simulink model "MX_Gateway"  (Simulink 9.0)
settings: Solver=FixedStepDiscrete  StartTime=0.0  StopTime=100  FixedStep=0.01  RelTol=1e-3
values from neighbouring files (symbols this model uses):
  ACTIVE = Bobcat.Parameter; CreateDefine( ACTIVE                         ,1                          ,'Define' ,'defines.h' )    [defines.m]
  ACTIVE_MODE_ONE = Bobcat.Parameter; CreateDefine( ACTIVE_MODE_ONE                ,19                         ,'Define' ,'defines.h' )    [defines.m]
  AUTHENTICATED = Bobcat.Parameter; CreateDefine( AUTHENTICATED                  ,1                          ,'Define' ,'defines.h' )    [defines.m]
  AUX_EMEA_CONTROLS = Bobcat.Parameter; CreateDefine( AUX_EMEA_CONTROLS  ,1   ,'Define' ,'defines.h' )    [enums.m]
  AUX_FLOW_HIGH = Bobcat.Parameter; CreateDefine( AUX_FLOW_HIGH           ,4  ,'Define' ,'defines.h' )    [enums.m]
  AUX_FLOW_INACTIVE = Bobcat.Parameter; CreateDefine( AUX_FLOW_INACTIVE       ,0  ,'Define' ,'defines.h' )    [enums.m]
  AUX_FLOW_LOW = Bobcat.Parameter; CreateDefine( AUX_FLOW_LOW            ,1  ,'Define' ,'defines.h' )    [enums.m]
  AUX_FLOW_MEDIUM = Bobcat.Parameter; CreateDefine( AUX_FLOW_MEDIUM         ,2  ,'Define' ,'defines.h' )    [enums.m]
  AUX_FLOW_STANDARD = Bobcat.Parameter; CreateDefine( AUX_FLOW_STANDARD       ,3  ,'Define' ,'defines.h' )    [enums.m]
  AUX_NON_FUNCTIONAL = Bobcat.Parameter; CreateDefine( AUX_NON_FUNCTIONAL ,255 ,'Define' ,'defines.h' )    [enums.m]
  BASE_ACTIVE = Bobcat.Parameter; CreateDefine( BASE_ACTIVE                    ,253                        ,'Define' ,'defines.h' )    [defines.m]
  BASE_DETENT = Bobcat.Parameter; CreateDefine( BASE_DETENT                    ,1                          ,'Define' ,'defines.h' )    [defines.m]
  BEEP_NONE = Bobcat.Parameter; CreateDefine( BEEP_NONE                      ,255                        ,'Define' ,'defines.h' )    [defines.m]
  CD_AIR_FILTER_DEBOUNCE_NORMAL = Bobcat.Parameter; CreateAliasCD( CD_AIR_FILTER_DEBOUNCE_NORMAL                        ,uint16(500)                ,'ConstantDataPointer' ,'CONSTANT_DATA' ,'AIR_FILTER_DEBOUNCE_NORMAL')    [cdata.m]    (also in hc12_cdata.m; m4_cdata.m)
  CD_AIR_FILTER_DEBOUNCE_NOT_CONNECTED = Bobcat.Parameter; CreateAliasCD( CD_AIR_FILTER_DEBOUNCE_NOT_CONNECTED                 ,uint16(3000)               ,'ConstantDataPointer' ,'CONSTANT_DATA' ,'AIR_FILTER_DEBOUNCE_NOT_CONNECTED')    [cdata.m]    (also in hc12_cdata.m; m4_cdata.m)
  CD_AIR_FILTER_DEBOUNCE_PLUGGED = Bobcat.Parameter; CreateAliasCD( CD_AIR_FILTER_DEBOUNCE_PLUGGED                       ,uint16(3000)               ,'ConstantDataPointer' ,'CONSTANT_DATA' ,'AIR_FILTER_DEBOUNCE_PLUGGED')    [cdata.m]    (also in hc12_cdata.m; m4_cdata.m)
  CD_AIR_FILTER_FUNCTION = Bobcat.Parameter; CreateAliasCD( CD_AIR_FILTER_FUNCTION                               ,uint8(255)                 ,'ConstantDataPointer' ,'CONSTANT_DATA' ,'AIR_FILTER_FUNCTION')    [cdata.m]    (also in hc12_cdata.m; m4_cdata.m)
  CD_AIR_FILTER_PLUGGED_WEIGHT = Bobcat.Parameter; CreateAliasCD( CD_AIR_FILTER_PLUGGED_WEIGHT                         ,uint8(2)                   ,'ConstantDataPointer' ,'CONSTANT_DATA' ,'AIR_FILTER_PLUGGED_WEIGHT')    [cdata.m]    (also in hc12_cdata.m; m4_cdata.m)
  CD_AIR_FILTER_UNPLUGGED_WEIGHT = Bobcat.Parameter; CreateAliasCD( CD_AIR_FILTER_UNPLUGGED_WEIGHT                       ,uint8(1)                   ,'ConstantDataPointer' ,'CONSTANT_DATA' ,'AIR_FILTER_UNPLUGGED_WEIGHT')    [cdata.m]    (also in hc12_cdata.m; m4_cdata.m)
  CD_AUTOIDLE_ARMBAR_DELAY = Bobcat.Parameter; CreateAliasCD( CD_AUTOIDLE_ARMBAR_DELAY                             ,uint8(2)                   ,'ConstantDataPointer' ,'CONSTANT_DATA' ,'AUTOIDLE_ARMBAR_DELAY')    [cdata.m]    (also in hc12_cdata.m; m4_cdata.m)
  CD_AUTOIDLE_FUNCTION = Bobcat.Parameter; CreateAliasCD( CD_AUTOIDLE_FUNCTION                                 ,uint8(255)                 ,'ConstantDataPointer' ,'CONSTANT_DATA' ,'AUTOIDLE_FUNCTION')    [cdata.m]    (also in hc12_cdata.m; m4_cdata.m)
  CD_AUTOIDLE_IDLE_DELAY = Bobcat.Parameter; CreateAliasCD( CD_AUTOIDLE_IDLE_DELAY                               ,uint8(40)                  ,'ConstantDataPointer' ,'CONSTANT_DATA' ,'AUTOIDLE_IDLE_DELAY')    [cdata.m]    (also in hc12_cdata.m; m4_cdata.m)
  CD_AUTOIDLE_LOW_IDLE_START = Bobcat.Parameter; CreateAliasCD( CD_AUTOIDLE_LOW_IDLE_START                           ,uint8(255)                 ,'ConstantDataPointer' ,'CONSTANT_DATA' ,'AUTOIDLE_LOW_IDLE_START')    [cdata.m]    (also in hc12_cdata.m; m4_cdata.m)
  CD_AUTOIDLE_PRESSURE_OFF = Bobcat.Parameter; CreateAliasCD( CD_AUTOIDLE_PRESSURE_OFF                             ,uint16(300)                ,'ConstantDataPointer' ,'CONSTANT_DATA' ,'AUTOIDLE_PRESSURE_OFF')    [cdata.m]    (also in hc12_cdata.m; m4_cdata.m)
  CD_AUTOIDLE_PRESSURE_ON = Bobcat.Parameter; CreateAliasCD( CD_AUTOIDLE_PRESSURE_ON                              ,uint16(270)                ,'ConstantDataPointer' ,'CONSTANT_DATA' ,'AUTOIDLE_PRESSURE_ON')    [cdata.m]    (also in hc12_cdata.m; m4_cdata.m)
  CD_AUTOSHIFT_FUNCTION = Bobcat.Parameter; CreateAliasCD( CD_AUTOSHIFT_FUNCTION                                ,uint8(255)                 ,'ConstantDataPointer' ,'CONSTANT_DATA' ,'AUTOSHIFT_FUNCTION')    [cdata.m]    (also in hc12_cdata.m; m4_cdata.m)
  CD_AUTOSHIFT_PHIGH1 = Bobcat.Parameter; CreateAliasCD( CD_AUTOSHIFT_PHIGH1                                  ,uint16(205)                ,'ConstantDataPointer' ,'CONSTANT_DATA' ,'AUTOSHIFT_PHIGH1')    [cdata.m]    (also in hc12_cdata.m; m4_cdata.m)
  CD_AUTOSHIFT_PHIGH2 = Bobcat.Parameter; CreateAliasCD( CD_AUTOSHIFT_PHIGH2                                  ,uint16(193)                ,'ConstantDataPointer' ,'CONSTANT_DATA' ,'AUTOSHIFT_PHIGH2')    [cdata.m]    (also in hc12_cdata.m; m4_cdata.m)
  CD_AUTOSHIFT_PHIGH_TEMP1 = Bobcat.Parameter; CreateAliasCD( CD_AUTOSHIFT_PHIGH_TEMP1                             ,int16(70)                  ,'ConstantDataPointer' ,'CONSTANT_DATA' ,'AUTOSHIFT_PHIGH_TEMP1')    [cdata.m]    (also in hc12_cdata.m; m4_cdata.m)
  CD_AUTOSHIFT_PHIGH_TEMP2 = Bobcat.Parameter; CreateAliasCD( CD_AUTOSHIFT_PHIGH_TEMP2                             ,int16(160)                 ,'ConstantDataPointer' ,'CONSTANT_DATA' ,'AUTOSHIFT_PHIGH_TEMP2')    [cdata.m]    (also in hc12_cdata.m; m4_cdata.m)
  CD_AUTOSHIFT_PLOW1 = Bobcat.Parameter; CreateAliasCD( CD_AUTOSHIFT_PLOW1                                   ,uint16(130)                ,'ConstantDataPointer' ,'CONSTANT_DATA' ,'AUTOSHIFT_PLOW1')    [cdata.m]    (also in hc12_cdata.m; m4_cdata.m)
  CD_AUTOSHIFT_PLOW2 = Bobcat.Parameter; CreateAliasCD( CD_AUTOSHIFT_PLOW2                                   ,uint16(100)                ,'ConstantDataPointer' ,'CONSTANT_DATA' ,'AUTOSHIFT_PLOW2')    [cdata.m]    (also in hc12_cdata.m; m4_cdata.m)
  CD_AUTOSHIFT_PLOW_TEMP1 = Bobcat.Parameter; CreateAliasCD( CD_AUTOSHIFT_PLOW_TEMP1                              ,int16(20)                  ,'ConstantDataPointer' ,'CONSTANT_DATA' ,'AUTOSHIFT_PLOW_TEMP1')    [cdata.m]    (also in hc12_cdata.m; m4_cdata.m)
  CD_AUTOSHIFT_PLOW_TEMP2 = Bobcat.Parameter; CreateAliasCD( CD_AUTOSHIFT_PLOW_TEMP2                              ,int16(80)                  ,'ConstantDataPointer' ,'CONSTANT_DATA' ,'AUTOSHIFT_PLOW_TEMP2')    [cdata.m]    (also in hc12_cdata.m; m4_cdata.m)
  CD_AUTOSHIFT_TRAVEL_DELAY = Bobcat.Parameter; CreateAliasCD( CD_AUTOSHIFT_TRAVEL_DELAY                            ,uint8(32)                  ,'ConstantDataPointer' ,'CONSTANT_DATA' ,'AUTOSHIFT_TRAVEL_DELAY')    [cdata.m]    (also in hc12_cdata.m; m4_cdata.m)
  CD_AUX2_CRACKPOINT_CURRENT = Bobcat.Parameter; CreateAliasCD( CD_AUX2_CRACKPOINT_CURRENT                           ,fi(0,0,16,12,'hex','0c28') ,'ConstantDataPointer' ,'CONSTANT_DATA' ,'AUX2_CRACKPOINT_CURRENT')    [cdata.m]    (also in hc12_cdata.m; m4_cdata.m)
  CD_AUX2_FLOW_LOW_FUNCTION = Bobcat.Parameter; CreateAliasCD( CD_AUX2_FLOW_LOW_FUNCTION                            ,uint8(255)                 ,'ConstantDataPointer' ,'CONSTANT_DATA' ,'AUX2_FLOW_LOW_FUNCTION')    [cdata.m]    (also in hc12_cdata.m; m4_cdata.m)
  CD_AUX2_FLOW_MED_FUNCTION = Bobcat.Parameter; CreateAliasCD( CD_AUX2_FLOW_MED_FUNCTION                            ,uint8(255)                 ,'ConstantDataPointer' ,'CONSTANT_DATA' ,'AUX2_FLOW_MED_FUNCTION')    [cdata.m]    (also in hc12_cdata.m; m4_cdata.m)
  CD_AUX2_HI_FLOW_PERCENT = Bobcat.Parameter; CreateAliasCD( CD_AUX2_HI_FLOW_PERCENT                              ,fi(0,0,16,16,'hex','ffff') ,'ConstantDataPointer' ,'CONSTANT_DATA' ,'AUX2_HI_FLOW_PERCENT')    [cdata.m]    (also in hc12_cdata.m; m4_cdata.m)
  CD_AUX2_LOW_FLOW_PERCENT = Bobcat.Parameter; CreateAliasCD( CD_AUX2_LOW_FLOW_PERCENT                             ,fi(0,0,16,16,'hex','7333') ,'ConstantDataPointer' ,'CONSTANT_DATA' ,'AUX2_LOW_FLOW_PERCENT')    [cdata.m]    (also in hc12_cdata.m; m4_cdata.m)
  CD_AUX2_MAX_FLOW_CURRENT = Bobcat.Parameter; CreateAliasCD( CD_AUX2_MAX_FLOW_CURRENT                             ,fi(0,0,16,12,'hex','14cc') ,'ConstantDataPointer' ,'CONSTANT_DATA' ,'AUX2_MAX_FLOW_CURRENT')    [cdata.m]    (also in hc12_cdata.m; m4_cdata.m)
  CD_AUX2_MED_FLOW_PERCENT = Bobcat.Parameter; CreateAliasCD( CD_AUX2_MED_FLOW_PERCENT                             ,fi(0,0,16,16,'hex','9999') ,'ConstantDataPointer' ,'CONSTANT_DATA' ,'AUX2_MED_FLOW_PERCENT')    [cdata.m]    (also in hc12_cdata.m; m4_cdata.m)
  CD_AUX_CRACKPOINT_CURRENT = Bobcat.Parameter; CreateAliasCD( CD_AUX_CRACKPOINT_CURRENT                            ,fi(0,0,16,12,'hex','0000') ,'ConstantDataPointer' ,'CONSTANT_DATA' ,'AUX_CRACKPOINT_CURRENT')    [cdata.m]    (also in hc12_cdata.m; m4_cdata.m)
  CD_AUX_DETENT_FLASH_TIME = Bobcat.Parameter; CreateAliasCD( CD_AUX_DETENT_FLASH_TIME                             ,uint16(72)                 ,'ConstantDataPointer' ,'CONSTANT_DATA' ,'AUX_DETENT_FLASH_TIME')    [cdata.m]    (also in hc12_cdata.m; m4_cdata.m)
  CD_AUX_DETENT_HOLD_TIME = Bobcat.Parameter; CreateAliasCD( CD_AUX_DETENT_HOLD_TIME                              ,uint16(100)                ,'ConstantDataPointer' ,'CONSTANT_DATA' ,'AUX_DETENT_HOLD_TIME')    [cdata.m]    (also in hc12_cdata.m; m4_cdata.m)
  CD_AUX_FULL_ON_AUX1 = Bobcat.Parameter; CreateAliasCD( CD_AUX_FULL_ON_AUX1                                  ,fi(0,0,16,12,'hex','10cd') ,'ConstantDataPointer' ,'CONSTANT_DATA' ,'AUX_FULL_ON_AUX1')    [cdata.m]    (also in hc12_cdata.m; m4_cdata.m)
  CD_AUX_FULL_ON_AUX2 = Bobcat.Parameter; CreateAliasCD( CD_AUX_FULL_ON_AUX2                                  ,fi(0,0,16,12,'hex','139d') ,'ConstantDataPointer' ,'CONSTANT_DATA' ,'AUX_FULL_ON_AUX2')    [cdata.m]    (also in hc12_cdata.m; m4_cdata.m)
  CD_AUX_FULL_ON_AUX3 = Bobcat.Parameter; CreateAliasCD( CD_AUX_FULL_ON_AUX3                                  ,fi(0,0,16,12,'hex','17d7') ,'ConstantDataPointer' ,'CONSTANT_DATA' ,'AUX_FULL_ON_AUX3')    [cdata.m]    (also in hc12_cdata.m; m4_cdata.m)
  CD_AUX_FUNCTION = Bobcat.Parameter; CreateAliasCD( CD_AUX_FUNCTION                                      ,uint8(0)                   ,'ConstantDataPointer' ,'CONSTANT_DATA' ,'AUX_FUNCTION')    [cdata.m]    (also in hc12_cdata.m; m4_cdata.m)
  CD_AUX_RATE_LIMIT_CRACKPOINT = Bobcat.Parameter; CreateAliasCD( CD_AUX_RATE_LIMIT_CRACKPOINT                         ,fi(0,0,16,12,'hex','0800') ,'ConstantDataPointer' ,'CONSTANT_DATA' ,'AUX_RATE_LIMIT_CRACKPOINT')    [cdata.m]    (also in hc12_cdata.m; m4_cdata.m)
  CD_AUX_RATE_LIMIT_CRACKPOINT_RATE = Bobcat.Parameter; CreateAliasCD( CD_AUX_RATE_LIMIT_CRACKPOINT_RATE                    ,fi(0,1,32,12,'int',102400) ,'ConstantDataPointer' ,'CONSTANT_DATA' ,'AUX_RATE_LIMIT_CRACKPOINT_RATE')    [cdata.m]    (also in hc12_cdata.m; m4_cdata.m)
  CD_AUX_RATE_LIMIT_FUNCTION = Bobcat.Parameter; CreateAliasCD( CD_AUX_RATE_LIMIT_FUNCTION                           ,uint8(255)                 ,'ConstantDataPointer' ,'CONSTANT_DATA' ,'AUX_RATE_LIMIT_FUNCTION')    [cdata.m]    (also in hc12_cdata.m; m4_cdata.m)
  CD_AUX_RATE_LIMIT_MAX_ENG_SPD = Bobcat.Parameter; CreateAliasCD( CD_AUX_RATE_LIMIT_MAX_ENG_SPD                        ,uint16(1600)               ,'ConstantDataPointer' ,'CONSTANT_DATA' ,'AUX_RATE_LIMIT_MAX_ENG_SPD')    [cdata.m]    (also in hc12_cdata.m; m4_cdata.m)
  CD_AUX_RATE_LIMIT_MIN_ENG_SPD = Bobcat.Parameter; CreateAliasCD( CD_AUX_RATE_LIMIT_MIN_ENG_SPD                        ,uint16(100)                ,'ConstantDataPointer' ,'CONSTANT_DATA' ,'AUX_RATE_LIMIT_MIN_ENG_SPD')    [cdata.m]    (also in hc12_cdata.m; m4_cdata.m)
  CD_AUX_RATE_LIMIT_NORMAL_RATE = Bobcat.Parameter; CreateAliasCD( CD_AUX_RATE_LIMIT_NORMAL_RATE                        ,fi(0,1,32,12,'int',2048)   ,'ConstantDataPointer' ,'CONSTANT_DATA' ,'AUX_RATE_LIMIT_NORMAL_RATE')    [cdata.m]    (also in hc12_cdata.m; m4_cdata.m)
  CD_AUXPOT_CAL_DEADBAND_HIGH = Bobcat.Parameter; CreateAliasCD( CD_AUXPOT_CAL_DEADBAND_HIGH                          ,uint16(574)                ,'ConstantDataPointer' ,'CONSTANT_DATA' ,'AUXPOT_CAL_DEADBAND_HIGH')    [cdata.m]    (also in hc12_cdata.m; m4_cdata.m)
  CD_AUXPOT_CAL_DEADBAND_LOW = Bobcat.Parameter; CreateAliasCD( CD_AUXPOT_CAL_DEADBAND_LOW                           ,uint16(424)                ,'ConstantDataPointer' ,'CONSTANT_DATA' ,'AUXPOT_CAL_DEADBAND_LOW')    [cdata.m]    (also in hc12_cdata.m; m4_cdata.m)
  CD_AUXPOT_CENTER_RATIO = Bobcat.Parameter; CreateAliasCD( CD_AUXPOT_CENTER_RATIO                               ,uint16(500)                ,'ConstantDataPointer' ,'CONSTANT_DATA' ,'AUXPOT_CENTER_RATIO')    [cdata.m]    (also in hc12_cdata.m; m4_cdata.m)
  CD_AUXPOT_DEADBAND = Bobcat.Parameter; CreateAliasCD( CD_AUXPOT_DEADBAND                                   ,uint8(38)                  ,'ConstantDataPointer' ,'CONSTANT_DATA' ,'AUXPOT_DEADBAND')    [cdata.m]    (also in hc12_cdata.m; m4_cdata.m)
  CD_AUXPOT_FUNCTION = Bobcat.Parameter; CreateAliasCD( CD_AUXPOT_FUNCTION                                   ,uint8(0)                   ,'ConstantDataPointer' ,'CONSTANT_DATA' ,'AUXPOT_FUNCTION')    [cdata.m]    (also in hc12_cdata.m; m4_cdata.m)
  CD_AUXPOT_RATIO_GAIN = Bobcat.Parameter; CreateAliasCD( CD_AUXPOT_RATIO_GAIN                                 ,uint16(1000)               ,'ConstantDataPointer' ,'CONSTANT_DATA' ,'AUXPOT_RATIO_GAIN')    [cdata.m]    (also in hc12_cdata.m; m4_cdata.m)
  CD_CAN_THROTTLE_FUNCTION = Bobcat.Parameter; CreateAliasCD( CD_CAN_THROTTLE_FUNCTION                             ,uint8(255)                 ,'ConstantDataPointer' ,'CONSTANT_DATA' ,'CAN_THROTTLE_FUNCTION')    [cdata.m]    (also in hc12_cdata.m; m4_cdata.m)
  CD_CAN_THROTTLE_REVERSE = Bobcat.Parameter; CreateAliasCD( CD_CAN_THROTTLE_REVERSE                              ,uint8(255)                 ,'ConstantDataPointer' ,'CONSTANT_DATA' ,'CAN_THROTTLE_REVERSE')    [cdata.m]    (also in hc12_cdata.m; m4_cdata.m)
  CD_CONSOLE_DEBOUNCE_ABSENT = Bobcat.Parameter; CreateAliasCD( CD_CONSOLE_DEBOUNCE_ABSENT                           ,uint16(12)                 ,'ConstantDataPointer' ,'CONSTANT_DATA' ,'CONSOLE_DEBOUNCE_ABSENT')    [cdata.m]    (also in hc12_cdata.m; m4_cdata.m)
  CD_CONSOLE_DEBOUNCE_OORH = Bobcat.Parameter; CreateAliasCD( CD_CONSOLE_DEBOUNCE_OORH                             ,uint16(12)                 ,'ConstantDataPointer' ,'CONSTANT_DATA' ,'CONSOLE_DEBOUNCE_OORH')    [cdata.m]    (also in hc12_cdata.m; m4_cdata.m)
  CD_CONSOLE_DEBOUNCE_OORL = Bobcat.Parameter; CreateAliasCD( CD_CONSOLE_DEBOUNCE_OORL                             ,uint16(12)                 ,'ConstantDataPointer' ,'CONSTANT_DATA' ,'CONSOLE_DEBOUNCE_OORL')    [cdata.m]    (also in hc12_cdata.m; m4_cdata.m)
  CD_CONSOLE_DEBOUNCE_PRESENT = Bobcat.Parameter; CreateAliasCD( CD_CONSOLE_DEBOUNCE_PRESENT                          ,uint16(12)                 ,'ConstantDataPointer' ,'CONSTANT_DATA' ,'CONSOLE_DEBOUNCE_PRESENT')    [cdata.m]    (also in hc12_cdata.m; m4_cdata.m)
  CD_CONSOLE_FUNCTION = Bobcat.Parameter; CreateAliasCD( CD_CONSOLE_FUNCTION                                  ,uint8(0)                   ,'ConstantDataPointer' ,'CONSTANT_DATA' ,'CONSOLE_FUNCTION')    [cdata.m]    (also in hc12_cdata.m; m4_cdata.m)
  CD_CONSOLE_OORH = Bobcat.Parameter; CreateAliasCD( CD_CONSOLE_OORH                                      ,uint16(740)                ,'ConstantDataPointer' ,'CONSTANT_DATA' ,'CONSOLE_OORH')    [cdata.m]    (also in hc12_cdata.m; m4_cdata.m)
  CD_CONSOLE_OORL = Bobcat.Parameter; CreateAliasCD( CD_CONSOLE_OORL                                      ,uint16(10)                 ,'ConstantDataPointer' ,'CONSTANT_DATA' ,'CONSOLE_OORL')    [cdata.m]    (also in hc12_cdata.m; m4_cdata.m)
  CD_CONSOLE_PRESENT_HIGH = Bobcat.Parameter; CreateAliasCD( CD_CONSOLE_PRESENT_HIGH                              ,uint16(515)                ,'ConstantDataPointer' ,'CONSTANT_DATA' ,'CONSOLE_PRESENT_HIGH')    [cdata.m]    (also in hc12_cdata.m; m4_cdata.m)
  CD_CONSOLE_PRESENT_LOW = Bobcat.Parameter; CreateAliasCD( CD_CONSOLE_PRESENT_LOW                               ,uint16(375)                ,'ConstantDataPointer' ,'CONSTANT_DATA' ,'CONSOLE_PRESENT_LOW')    [cdata.m]    (also in hc12_cdata.m; m4_cdata.m)
  CD_DELUXE_GEN5_5_FUNCTION = Bobcat.Parameter; CreateAliasCD( CD_DELUXE_GEN5_5_FUNCTION                            ,uint8(255)                 ,'ConstantDataPointer' ,'CONSTANT_DATA' ,'DELUXE_GEN5_5_FUNCTION')    [cdata.m]    (also in hc12_cdata.m; m4_cdata.m)
  CD_DELUXE_GEN5_FUNCTION = Bobcat.Parameter; CreateAliasCD( CD_DELUXE_GEN5_FUNCTION                              ,uint8(255)                 ,'ConstantDataPointer' ,'CONSTANT_DATA' ,'DELUXE_GEN5_FUNCTION')    [cdata.m]    (also in hc12_cdata.m; m4_cdata.m)
  CD_DERATE_ENGINE_SPEED_DEBOUNCE_HIGH = Bobcat.Parameter; CreateAliasCD( CD_DERATE_ENGINE_SPEED_DEBOUNCE_HIGH                 ,uint16(200)                ,'ConstantDataPointer' ,'CONSTANT_DATA' ,'DERATE_ENGINE_SPEED_DEBOUNCE_HIGH')    [cdata.m]    (also in hc12_cdata.m; m4_cdata.m)
  CD_DERATE_ENGINE_SPEED_DEBOUNCE_SHUTDOWN = Bobcat.Parameter; CreateAliasCD( CD_DERATE_ENGINE_SPEED_DEBOUNCE_SHUTDOWN             ,uint16(1000)               ,'ConstantDataPointer' ,'CONSTANT_DATA' ,'DERATE_ENGINE_SPEED_DEBOUNCE_SHUTDOWN')    [cdata.m]    (also in hc12_cdata.m; m4_cdata.m)
  CD_DERATE_ENGINE_SPEED_HIGH_OFFSET = Bobcat.Parameter; CreateAliasCD( CD_DERATE_ENGINE_SPEED_HIGH_OFFSET                   ,uint16(200)                ,'ConstantDataPointer' ,'CONSTANT_DATA' ,'DERATE_ENGINE_SPEED_HIGH_OFFSET')    [cdata.m]    (also in hc12_cdata.m; m4_cdata.m)
  CD_DERATE_METHOD = Bobcat.Parameter; CreateAliasCD( CD_DERATE_METHOD                                     ,uint8(0)                   ,'ConstantDataPointer' ,'CONSTANT_DATA' ,'DERATE_METHOD')    [cdata.m]    (also in hc12_cdata.m; m4_cdata.m)
  CD_DUMP_VALVE_FUNCTION = Bobcat.Parameter; CreateAliasCD( CD_DUMP_VALVE_FUNCTION                               ,uint8(255)                 ,'ConstantDataPointer' ,'CONSTANT_DATA' ,'DUMP_VALVE_FUNCTION')    [cdata.m]    (also in hc12_cdata.m; m4_cdata.m)
  CD_DUMP_VALVE_ON_CURRENT = Bobcat.Parameter; CreateAliasCD( CD_DUMP_VALVE_ON_CURRENT                             ,fi(0,0,16,12,'hex','ffff') ,'ConstantDataPointer' ,'CONSTANT_DATA' ,'DUMP_VALVE_ON_CURRENT')    [cdata.m]    (also in hc12_cdata.m; m4_cdata.m)
  CD_ECO_MODE_THROTTLE_REDUCTION = Bobcat.Parameter; CreateAliasCD( CD_ECO_MODE_THROTTLE_REDUCTION                       ,uint16(850)                ,'ConstantDataPointer' ,'CONSTANT_DATA' ,'ECO_MODE_THROTTLE_REDUCTION')    [cdata.m]    (also in hc12_cdata.m; m4_cdata.m)
  CD_ECT_FILTER_GAIN = Bobcat.Parameter; CreateAliasCD( CD_ECT_FILTER_GAIN                                   ,uint8(6)                   ,'ConstantDataPointer' ,'CONSTANT_DATA' ,'ECT_FILTER_GAIN')    [cdata.m]    (also in hc12_cdata.m; m4_cdata.m)
  CD_ECU_FUNCTION = Bobcat.Parameter; CreateAliasCD( CD_ECU_FUNCTION                                      ,uint8(255)                 ,'ConstantDataPointer' ,'CONSTANT_DATA' ,'ECU_FUNCTION')    [cdata.m]    (also in hc12_cdata.m; m4_cdata.m)
  CD_ECU_HOLD_DEBOUNCE_ERROR_ON = Bobcat.Parameter; CreateAliasCD( CD_ECU_HOLD_DEBOUNCE_ERROR_ON                        ,uint16(20)                 ,'ConstantDataPointer' ,'CONSTANT_DATA' ,'ECU_HOLD_DEBOUNCE_ERROR_ON')    [cdata.m]    (also in hc12_cdata.m; m4_cdata.m)
  CD_EMEA_CONTROLS_HOLD_TIME = Bobcat.Parameter; CreateAliasCD( CD_EMEA_CONTROLS_HOLD_TIME                           ,uint16(30)                 ,'ConstantDataPointer' ,'CONSTANT_DATA' ,'EMEA_CONTROLS_HOLD_TIME')    [cdata.m]    (also in hc12_cdata.m; m4_cdata.m)
  CD_EMEA_CONTROLS_OPTION_A = Bobcat.Parameter; CreateAliasCD( CD_EMEA_CONTROLS_OPTION_A                            ,uint8(255)                 ,'ConstantDataPointer' ,'CONSTANT_DATA' ,'EMEA_CONTROLS_OPTION_A')    [cdata.m]    (also in hc12_cdata.m; m4_cdata.m)
  CD_EMEA_CONTROLS_OPTION_C = Bobcat.Parameter; CreateAliasCD( CD_EMEA_CONTROLS_OPTION_C                            ,uint8(255)                 ,'ConstantDataPointer' ,'CONSTANT_DATA' ,'EMEA_CONTROLS_OPTION_C')    [cdata.m]    (also in hc12_cdata.m; m4_cdata.m)
  CD_EMEA_CONTROLS_REVERSE = Bobcat.Parameter; CreateAliasCD( CD_EMEA_CONTROLS_REVERSE                             ,uint8(255)                 ,'ConstantDataPointer' ,'CONSTANT_DATA' ,'EMEA_CONTROLS_REVERSE')    [cdata.m]    (also in hc12_cdata.m; m4_cdata.m)
  CD_EMEA_ELECTRIC_VERSION = Bobcat.Parameter; CreateAliasCD( CD_EMEA_ELECTRIC_VERSION                             ,uint8(0)                   ,'ConstantDataPointer' ,'CONSTANT_DATA' ,'EMEA_ELECTRIC_VERSION')    [cdata.m]    (also in hc12_cdata.m; m4_cdata.m)
  CD_EMEA_POT_CAL_DEADBAND_HIGH = Bobcat.Parameter; CreateAliasCD( CD_EMEA_POT_CAL_DEADBAND_HIGH                        ,uint16(574)                ,'ConstantDataPointer' ,'CONSTANT_DATA' ,'EMEA_POT_CAL_DEADBAND_HIGH')    [cdata.m]    (also in hc12_cdata.m; m4_cdata.m)
  CD_EMEA_POT_CAL_DEADBAND_LOW = Bobcat.Parameter; CreateAliasCD( CD_EMEA_POT_CAL_DEADBAND_LOW                         ,uint16(424)                ,'ConstantDataPointer' ,'CONSTANT_DATA' ,'EMEA_POT_CAL_DEADBAND_LOW')    [cdata.m]    (also in hc12_cdata.m; m4_cdata.m)
  CD_ENGINE_RPM_FACTOR = Bobcat.Parameter; CreateAliasCD( CD_ENGINE_RPM_FACTOR                                 ,uint8(35)                  ,'ConstantDataPointer' ,'CONSTANT_DATA' ,'ENGINE_RPM_FACTOR')    [cdata.m]    (also in hc12_cdata.m; m4_cdata.m)
  CD_ENGINE_SPEED_FILTER_FAST_VALUE = Bobcat.Parameter; CreateAliasCD( CD_ENGINE_SPEED_FILTER_FAST_VALUE                    ,uint8(1)                   ,'ConstantDataPointer' ,'CONSTANT_DATA' ,'ENGINE_SPEED_FILTER_FAST_VALUE')    [cdata.m]    (also in hc12_cdata.m; m4_cdata.m)
  CD_ENGINE_SPEED_FILTER_SLOW_VALUE = Bobcat.Parameter; CreateAliasCD( CD_ENGINE_SPEED_FILTER_SLOW_VALUE                    ,uint8(4)                   ,'ConstantDataPointer' ,'CONSTANT_DATA' ,'ENGINE_SPEED_FILTER_SLOW_VALUE')    [cdata.m]    (also in hc12_cdata.m; m4_cdata.m)
  CD_ENGINE_SPEED_RUNNING = Bobcat.Parameter; CreateAliasCD( CD_ENGINE_SPEED_RUNNING                              ,uint16(800)                ,'ConstantDataPointer' ,'CONSTANT_DATA' ,'ENGINE_SPEED_RUNNING')    [cdata.m]    (also in hc12_cdata.m; m4_cdata.m)
  CD_ENGINE_START_DELAY_DEBOUNCE_RPM_NO_SIGNAL = Bobcat.Parameter; CreateAliasCD( CD_ENGINE_START_DELAY_DEBOUNCE_RPM_NO_SIGNAL         ,uint16(0)                  ,'ConstantDataPointer' ,'CONSTANT_DATA' ,'ENGINE_START_DELAY_DEBOUNCE_RPM_NO_SIGNAL')    [cdata.m]    (also in hc12_cdata.m; m4_cdata.m)
  CD_ENGINE_START_DELAY_OFF_TIME = Bobcat.Parameter; CreateAliasCD( CD_ENGINE_START_DELAY_OFF_TIME                       ,uint16(0)                  ,'ConstantDataPointer' ,'CONSTANT_DATA' ,'ENGINE_START_DELAY_OFF_TIME')    [cdata.m]    (also in hc12_cdata.m; m4_cdata.m)
  CD_ENGINE_START_DELAY_RPM_NO_SIGNAL = Bobcat.Parameter; CreateAliasCD( CD_ENGINE_START_DELAY_RPM_NO_SIGNAL                  ,uint16(0)                  ,'ConstantDataPointer' ,'CONSTANT_DATA' ,'ENGINE_START_DELAY_RPM_NO_SIGNAL')    [cdata.m]    (also in hc12_cdata.m; m4_cdata.m)
  CD_EOP_DEBOUNCE_EXTREMELY_LOW = Bobcat.Parameter; CreateAliasCD( CD_EOP_DEBOUNCE_EXTREMELY_LOW                        ,uint16(50)                 ,'ConstantDataPointer' ,'CONSTANT_DATA' ,'EOP_DEBOUNCE_EXTREMELY_LOW')    [cdata.m]    (also in hc12_cdata.m; m4_cdata.m)
  CD_EOP_DEBOUNCE_NORMAL = Bobcat.Parameter; CreateAliasCD( CD_EOP_DEBOUNCE_NORMAL                               ,uint16(50)                 ,'ConstantDataPointer' ,'CONSTANT_DATA' ,'EOP_DEBOUNCE_NORMAL')    [cdata.m]    (also in hc12_cdata.m; m4_cdata.m)
  CD_EOP_DEBOUNCE_SHUTDOWN = Bobcat.Parameter; CreateAliasCD( CD_EOP_DEBOUNCE_SHUTDOWN                             ,uint16(100)                ,'ConstantDataPointer' ,'CONSTANT_DATA' ,'EOP_DEBOUNCE_SHUTDOWN')    [cdata.m]    (also in hc12_cdata.m; m4_cdata.m)
  CD_EOP_FILTER_VALUE = Bobcat.Parameter; CreateAliasCD( CD_EOP_FILTER_VALUE                                  ,uint8(3)                   ,'ConstantDataPointer' ,'CONSTANT_DATA' ,'EOP_FILTER_VALUE')    [cdata.m]    (also in hc12_cdata.m; m4_cdata.m)
  CD_EOP_FUNCTION = Bobcat.Parameter; CreateAliasCD( CD_EOP_FUNCTION                                      ,uint8(1)                   ,'ConstantDataPointer' ,'CONSTANT_DATA' ,'EOP_FUNCTION')    [cdata.m]    (also in hc12_cdata.m; m4_cdata.m)
  CD_EOP_SWITCH_GAUGE_VALUE = Bobcat.Parameter; CreateAliasCD( CD_EOP_SWITCH_GAUGE_VALUE                            ,uint16(125)                ,'ConstantDataPointer' ,'CONSTANT_DATA' ,'EOP_SWITCH_GAUGE_VALUE')    [cdata.m]    (also in hc12_cdata.m; m4_cdata.m)
  CD_EOP_SWITCH_LOW = Bobcat.Parameter; CreateAliasCD( CD_EOP_SWITCH_LOW                                    ,uint16(50)                 ,'ConstantDataPointer' ,'CONSTANT_DATA' ,'EOP_SWITCH_LOW')    [cdata.m]    (also in hc12_cdata.m; m4_cdata.m)
  CD_EOP_SWITCH_PRESSURE_VALUE = Bobcat.Parameter; CreateAliasCD( CD_EOP_SWITCH_PRESSURE_VALUE                         ,uint16(40)                 ,'ConstantDataPointer' ,'CONSTANT_DATA' ,'EOP_SWITCH_PRESSURE_VALUE')    [cdata.m]    (also in hc12_cdata.m; m4_cdata.m)
  CD_EOT_DEBOUNCE_EXTREMELY_LOW = Bobcat.Parameter; CreateAliasCD( CD_EOT_DEBOUNCE_EXTREMELY_LOW                        ,uint16(2)                  ,'ConstantDataPointer' ,'CONSTANT_DATA' ,'EOT_DEBOUNCE_EXTREMELY_LOW')    [cdata.m]    (also in hc12_cdata.m; m4_cdata.m)
  CD_EOT_DEBOUNCE_LOW = Bobcat.Parameter; CreateAliasCD( CD_EOT_DEBOUNCE_LOW                                  ,uint16(2)                  ,'ConstantDataPointer' ,'CONSTANT_DATA' ,'EOT_DEBOUNCE_LOW')    [cdata.m]    (also in hc12_cdata.m; m4_cdata.m)
  CD_EOT_DEBOUNCE_NORMAL = Bobcat.Parameter; CreateAliasCD( CD_EOT_DEBOUNCE_NORMAL                               ,uint16(2)                  ,'ConstantDataPointer' ,'CONSTANT_DATA' ,'EOT_DEBOUNCE_NORMAL')    [cdata.m]    (also in hc12_cdata.m; m4_cdata.m)
  CD_EOT_EXTREMELY_LOW = Bobcat.Parameter; CreateAliasCD( CD_EOT_EXTREMELY_LOW                                 ,uint16(81)                 ,'ConstantDataPointer' ,'CONSTANT_DATA' ,'EOT_EXTREMELY_LOW')    [cdata.m]    (also in hc12_cdata.m; m4_cdata.m)
  CD_EOT_FUNCTION = Bobcat.Parameter; CreateAliasCD( CD_EOT_FUNCTION                                      ,uint8(255)                 ,'ConstantDataPointer' ,'CONSTANT_DATA' ,'EOT_FUNCTION')    [cdata.m]    (also in hc12_cdata.m; m4_cdata.m)
  CD_EOT_LOW = Bobcat.Parameter; CreateAliasCD( CD_EOT_LOW                                           ,uint16(90)                 ,'ConstantDataPointer' ,'CONSTANT_DATA' ,'EOT_LOW')    [cdata.m]    (also in hc12_cdata.m; m4_cdata.m)
  CD_EOT_TEMPERATURE_HYSTERESIS = Bobcat.Parameter; CreateAliasCD( CD_EOT_TEMPERATURE_HYSTERESIS                        ,uint16(2)                  ,'ConstantDataPointer' ,'CONSTANT_DATA' ,'EOT_TEMPERATURE_HYSTERESIS')    [cdata.m]    (also in hc12_cdata.m; m4_cdata.m)
  CD_FAST_TRAVEL_FUNCTION = Bobcat.Parameter; CreateAliasCD( CD_FAST_TRAVEL_FUNCTION                              ,uint8(255)                 ,'ConstantDataPointer' ,'CONSTANT_DATA' ,'FAST_TRAVEL_FUNCTION')    [cdata.m]    (also in hc12_cdata.m; m4_cdata.m)
  CD_FAST_TRAVEL_THROTTLE_DELAY = Bobcat.Parameter; CreateAliasCD( CD_FAST_TRAVEL_THROTTLE_DELAY                        ,uint16(10)                 ,'ConstantDataPointer' ,'CONSTANT_DATA' ,'FAST_TRAVEL_THROTTLE_DELAY')    [cdata.m]    (also in hc12_cdata.m; m4_cdata.m)
  CD_FAST_TRAVEL_THROTTLE_LIMIT = Bobcat.Parameter; CreateAliasCD( CD_FAST_TRAVEL_THROTTLE_LIMIT                        ,uint16(1000)               ,'ConstantDataPointer' ,'CONSTANT_DATA' ,'FAST_TRAVEL_THROTTLE_LIMIT')    [cdata.m]    (also in hc12_cdata.m; m4_cdata.m)
  CD_FLYWHEEL_TEETH = Bobcat.Parameter; CreateAliasCD( CD_FLYWHEEL_TEETH                                    ,uint8(83)                  ,'ConstantDataPointer' ,'CONSTANT_DATA' ,'FLYWHEEL_TEETH')    [cdata.m]    (also in hc12_cdata.m; m4_cdata.m)
  CD_FUEL_LEVEL_FILTER_FAST_VALUE = Bobcat.Parameter; CreateAliasCD( CD_FUEL_LEVEL_FILTER_FAST_VALUE                      ,uint8(5)                   ,'ConstantDataPointer' ,'CONSTANT_DATA' ,'FUEL_LEVEL_FILTER_FAST_VALUE')    [cdata.m]    (also in hc12_cdata.m; m4_cdata.m)
  CD_FUEL_LEVEL_FILTER_SLOW_VALUE = Bobcat.Parameter; CreateAliasCD( CD_FUEL_LEVEL_FILTER_SLOW_VALUE                      ,uint8(10)                  ,'ConstantDataPointer' ,'CONSTANT_DATA' ,'FUEL_LEVEL_FILTER_SLOW_VALUE')    [cdata.m]    (also in hc12_cdata.m; m4_cdata.m)
  CD_FUEL_PRIMARY_FEEDBACK_FUNCTION = Bobcat.Parameter; CreateAliasCD( CD_FUEL_PRIMARY_FEEDBACK_FUNCTION                    ,uint8(0)                   ,'ConstantDataPointer' ,'CONSTANT_DATA' ,'FUEL_PRIMARY_FEEDBACK_FUNCTION')    [cdata.m]    (also in hc12_cdata.m; m4_cdata.m)
  ... (+246 more)
undefined symbols (not defined in the file or in its folder): sigbuilder_block
undefined symbols (not defined in the file): CD_FUEL_RESOLUTION_TIMER
libraries: simulink x186, MX_Library x107
(part 26 of 48)

== stateflow chart MX_Gtwy_Control/Inputs/Console_Sensor/Console_Sensor ==
  state NON_FUNCTIONAL
    en:
    state_Console = NON_FUNCTIONAL;
  note: %wait for eeprom to be read since that effects machine Lock
  state STATE
    state NORMAL
      state ABSENT
        state DEBOUNCE_ABSENT
          en:
          debounceCount = 0;
          du:
          debounceCount++;
        state ABSENT
          en:
          state_Console = INACTIVE;
          SendMsg_BICSControl_Now();
        (default) -> DEBOUNCE_ABSENT
        DEBOUNCE_ABSENT -> STATE.NORMAL.ABSENT.ABSENT : [(debounceCount >= CD_CONSOLE_DEBOUNCE_ABSENT)...
            | || (state_Console == INACTIVE)]
      state PRESENT
        state DEBOUNCE_PRESENT
          en:
          debounceCount = 0;
          du:
          debounceCount++;
        state PRESENT
          en:
          state_Console = ACTIVE;
          SendMsg_BICSControl_Now();
        (default) -> DEBOUNCE_PRESENT
        DEBOUNCE_PRESENT -> STATE.NORMAL.PRESENT.PRESENT : [(debounceCount >= CD_CONSOLE_DEBOUNCE_PRESENT)...
            | || (state_Console == ACTIVE)]
      (default) -> STATE.NORMAL.ABSENT
      STATE.NORMAL.PRESENT -> STATE.NORMAL.ABSENT : [(rm_Console < CD_CONSOLE_PRESENT_LOW)...
          | || (rm_Console > CD_CONSOLE_PRESENT_HIGH)...
          | || (state_SensorSupply2 != NORMAL)...
          | || (state_SensorSupply1 != NORMAL)...
          | || (state_MachineLock == ACTIVE)]
      STATE.NORMAL.ABSENT -> STATE.NORMAL.PRESENT : [(rm_Console >= CD_CONSOLE_PRESENT_LOW) ...
          | && (rm_Console <= CD_CONSOLE_PRESENT_HIGH)...
          | && (state_SensorSupply2 == NORMAL)...
          | && (state_SensorSupply1 == NORMAL)...
          | && (state_MachineLock == INACTIVE)]
    state OORH
      state DEBOUNCE
        en:
        debounceCount = 0;
        du:
        debounceCount++;
      state OORH
        en:
        state_Console = OORH;
        SendMsg_BICSControl_Now();
      (default) -> STATE.OORH.DEBOUNCE
      STATE.OORH.DEBOUNCE -> STATE.OORH.OORH : [(debounceCount >= CD_CONSOLE_DEBOUNCE_OORH)]
    state OORL
      state DEBOUNCE
        en:
        debounceCount = 0;
        du:
        debounceCount++;
      state OORL
        en:
        state_Console = OORL;
        SendMsg_BICSControl_Now();
      (default) -> STATE.OORL.DEBOUNCE
      STATE.OORL.DEBOUNCE -> STATE.OORL.OORL : [(debounceCount >= CD_CONSOLE_DEBOUNCE_OORL)]
    (default) -> NORMAL
    STATE.OORH -> NORMAL : [((ain_Console< CD_CONSOLE_OORH)...
        | && (state_Console != OORH))...
        | || (state_SensorSupply2 != NORMAL)]
    STATE.OORL -> NORMAL : [((ain_Console > CD_CONSOLE_OORL)...
        | && (state_Console != OORL))...
        | || (state_SensorSupply2 != NORMAL)]
    NORMAL -> STATE.OORH [order 2] : [(ain_Console >= CD_CONSOLE_OORH)...
        | && (state_SensorSupply2 == NORMAL)]
    NORMAL -> STATE.OORL [order 1] : [(ain_Console <= CD_CONSOLE_OORL)...
        | && (state_SensorSupply2 == NORMAL)]
  (default) -> NON_FUNCTIONAL
  NON_FUNCTIONAL -> STATE : [(CD_CONSOLE_FUNCTION != NON_FUNCTIONAL)...
      | && (state_System == SYS_NORMAL)...
      | && (flag_InitEERead)]
  STATE -> NON_FUNCTIONAL : [(state_System != SYS_NORMAL)]
  data: state_Console output uint8; rm_Console input uint16; CD_CONSOLE_PRESENT_LOW parameter uint16; CD_CONSOLE_PRESENT_HIGH parameter uint16; CD_CONSOLE_FUNCTION parameter uint8; CD_CONSOLE_DEBOUNCE_PRESENT parameter uint16; CD_CONSOLE_DEBOUNCE_ABSENT parameter uint16; debounceCount local uint16 =0; CD_CONSOLE_OORH parameter uint16; CD_CONSOLE_DEBOUNCE_OORH parameter uint16; CD_CONSOLE_OORL parameter uint16; CD_CONSOLE_DEBOUNCE_OORL parameter uint16; ain_Console input uint16; state_SensorSupply1 input uint8; ACTIVE parameter uint8; INACTIVE parameter uint8; OORH parameter uint8; OORL parameter uint8; NON_FUNCTIONAL parameter uint8; state_SensorSupply2 input uint8; NORMAL parameter uint8; state_System input uint8; SYS_NORMAL parameter uint8; state_MachineLock input uint8; flag_InitEERead input boolean
  data rm_Console settings: description=Ratiometric value of the seatbar, calculated based on the sensor supply voltage and the analog voltage.

== stateflow chart MX_Gtwy_Control/System/Machine_Lock/Lock_Status ==
  state DELUXE_G55
    state LOCK
      en:state_MachineLock = ACTIVE;
    state UNLOCK
      en:state_MachineLock = INACTIVE;
    (default) -> DELUXE_G55.LOCK
    DELUXE_G55.LOCK -> DELUXE_G55.UNLOCK : [(state_System == SYS_NORMAL)...
        | && (state_CommDeluxeG55 == NORMAL)...
        | && (state_DeluxeG55 == DELUXE_G55_FUNCTION_UNLOCKED)...
        | && (auth_DeluxeG55 == AUTHENTICATED)]
  state NON_FUNCTIONAL
    en:
    state_MachineLock = ACTIVE;
  state KEYLESS_GENV_DELUXE
    state LOCK
      en:state_MachineLock = ACTIVE;
    state UNLOCK
      en:state_MachineLock = INACTIVE;
    (default) -> KEYLESS_GENV_DELUXE.LOCK
    KEYLESS_GENV_DELUXE.LOCK -> (junction 331) : [(flag_InitEERead)...
        | && (state_System == SYS_NORMAL)]
    (junction 331) -> KEYLESS_GENV_DELUXE.UNLOCK [order 1] : %no devices on machine that require locking
        | [!ee_Keypad && !ee_DeluxeG5...
        | && (CD_DELUXE_GEN5_5_FUNCTION == NON_FUNCTIONAL)]
    (junction 331) -> (junction 332) [order 2]
    (junction 332) -> KEYLESS_GENV_DELUXE.UNLOCK [order 1] : %No password lock
        | [ee_PasswordLock == INACTIVE]
    (junction 332) -> (junction 333) [order 2]
    (junction 333) -> KEYLESS_GENV_DELUXE.UNLOCK [order 1] : %Keyless Keypad installed, communicating and unlocked
        | [ee_Keypad && (state_KeylessPanel == NORMAL) && (state_KSLock == KS_UNLOCK)]
    (junction 333) -> (junction 334) [order 2]
    (junction 334) -> KEYLESS_GENV_DELUXE.UNLOCK : %Gen 5 Deluxe installed, communicating and unlocked
        | [ee_DeluxeG5 && (state_DeluxeG5 == NORMAL) && ddp_MachineLock ]
    KEYLESS_GENV_DELUXE.UNLOCK -> KEYLESS_GENV_DELUXE.LOCK : [((ee_Keypad == ACTIVE) && (state_KSLock == KS_LOCK))]
  (default) -> NON_FUNCTIONAL
  NON_FUNCTIONAL -> DELUXE_G55 [order 1] : [CD_DELUXE_GEN5_5_FUNCTION!= NON_FUNCTIONAL]
  NON_FUNCTIONAL -> KEYLESS_GENV_DELUXE [order 2]
  data: ddp_MachineLock input uint8; state_MachineLock output uint8; INACTIVE parameter uint8; ACTIVE parameter uint8; NORMAL parameter uint8; state_KeylessPanel input uint8; state_KSLock input uint8; state_System input uint8; SYS_NORMAL parameter uint8; KS_UNLOCK parameter uint8; KS_LOCK parameter uint8; state_DeluxeG5 input uint8; flag_InitEERead input boolean; ee_DeluxeG5 data_store_memory double; ee_PasswordLock data_store_memory double; ee_Keypad data_store_memory double; CD_DELUXE_GEN5_5_FUNCTION parameter uint8; NON_FUNCTIONAL parameter uint8; state_CommDeluxeG55 input uint8; auth_DeluxeG55 input uint8; state_DeluxeG55 input uint8; AUTHENTICATED parameter uint8; DELUXE_G55_FUNCTION_UNLOCKED parameter uint8

== matlab function MX_Gtwy_Control/Outputs/Aux_Hydraulics/Aux_Hydraulics/Aux_Hyd_Commands_Current_Control/Aux_Control_Logic ==
  data: state_AuxEnable input uint8; state_AuxPot input uint8; state_Detent input uint8; state_Engine input uint8; icmd_Pot input fixdt(0,16,12); ACTIVE_MODE_ONE parameter uint8; icmd_Base output fixdt(0,16,12); icmd_Rod output fixdt(0,16,12); BASE_ACTIVE parameter uint8; ROD_ACTIVE parameter uint8; BASE_DETENT parameter uint8; ROD_DETENT parameter uint8; ENGINE_CRANKING parameter uint8; icmd_Detent input fixdt(0,16,12); can_ACD_AuxCommand input uint8; can_AuxEnable_ACD input uint8; NON_FUNCTIONAL parameter uint8; CD_RACD_FUNCTION parameter uint8; ACDVarFlow local uint8; ACTIVE parameter uint8; cmd_AuxRelease input uint8; state_AuxFlow output uint8; INACTIVE parameter uint8
function [icmd_Base, icmd_Rod, state_AuxFlow] = fcn(state_AuxEnable, state_AuxPot, state_Detent, state_Engine, icmd_Pot, ACTIVE_MODE_ONE, BASE_ACTIVE, ROD_ACTIVE, BASE_DETENT, ROD_DETENT, ENGINE_CRANKING, icmd_Detent, can_ACD_AuxCommand, can_AuxEnable_ACD, NON_FUNCTIONAL, CD_RACD_FUNCTION, ACTIVE, cmd_AuxRelease, INACTIVE)

aVarTruthTableCondition_1 = false;
aVarTruthTableCondition_2 = false;
aVarTruthTableCondition_3 = false;
aVarTruthTableCondition_4 = false;
aVarTruthTableCondition_5 = false;
aVarTruthTableCondition_6 = false;
aVarTruthTableCondition_7 = false;
aVarTruthTableCondition_8 = false;
aVarTruthTableCondition_9 = false;
aVarTruthTableCondition_10 = false;
aVarTruthTableCondition_11 = false;
aVarTruthTableCondition_12 = false;
aVarTruthTableCondition_13 = false;


% Aux Enabled

aVarTruthTableCondition_1 = logical(state_AuxEnable == ACTIVE_MODE_ONE);

% Aux Pot - Base Active

aVarTruthTableCondition_2 = logical(state_AuxPot == BASE_ACTIVE);

% Aux Pot - Rod Active

aVarTruthTableCondition_3 = logical(state_AuxPot == ROD_ACTIVE);

% Base Detent

aVarTruthTableCondition_4 = logical(state_Detent == BASE_DETENT);

% Rod Detent

aVarTruthTableCondition_5 = logical(state_Detent == ROD_DETENT);

% ACD Functional

aVarTruthTableCondition_6 = logical(CD_RACD_FUNCTION ~= NON_FUNCTIONAL);

% ACD Active

aVarTruthTableCondition_7 = logical(can_AuxEnable_ACD == ACTIVE);

% ACD Base Active

aVarTruthTableCondition_8 = logical(can_ACD_AuxCommand == 1 || can_ACD_AuxCommand == 5);

% ACD Rod Active

aVarTruthTableCondition_9 = logical(can_ACD_AuxCommand == 4);

% ACD was in variable flow

aVarTruthTableCondition_10 = logical(ACDVarFlow > 0);

% Aux Release Rod

aVarTruthTableCondition_11 = logical(cmd_AuxRelease == ROD_ACTIVE);

% Aux Release Base

aVarTruthTableCondition_12 = logical(cmd_AuxRelease == BASE_ACTIVE);

% Engine Cranking

aVarTruthTableCondition_13 = logical(state_Engine == ENGINE_CRANKING);

if (aVarTruthTableCondition_13)
    A1();
elseif (~aVarTruthTableCondition_1 && ~aVarTruthTableCondition_7 && aVarTruthTableCondition_11 && ~aVarTruthTableCondition_12)
    A2();
elseif (~aVarTruthTableCondition_1 && ~aVarTruthTableCondition_7 && ~aVarTruthTableCondition_11 && aVarTruthTableCondition_12)
    A3();
elseif (aVarTruthTableCondition_1 && ~aVarTruthTableCondition_2 && ~aVarTruthTableCondition_3 && ~aVarTruthTableCondition_4 && aVarTruthTableCondition_5 && ~aVarTruthTableCondition_7 && ~aVarTruthTableCondition_11 && ~aVarTruthTableCondition_12)
    A2();
elseif (aVarTruthTableCondition_1 && ~aVarTruthTableCondition_2 && ~aVarTruthTableCondition_3 && aVarTruthTableCondition_4 && ~aVarTruthTableCondition_5 && ~aVarTruthTableCondition_7 && ~aVarTruthTableCondition_11 && ~aVarTruthTableCondition_12)
    A3();
elseif (aVarTruthTableCondition_1 && ~aVarTruthTableCondition_2 && aVarTruthTableCondition_3 && ~aVarTruthTableCondition_7 && ~aVarTruthTableCondition_11 && ~aVarTruthTableCondition_12)
    A4();
elseif (aVarTruthTableCondition_1 && aVarTruthTableCondition_2 && ~aVarTruthTableCondition_3 && ~aVarTruthTableCondition_7 && ~aVarTruthTableCondition_11 && ~aVarTruthTableCondition_12)
    A5();
elseif (aVarTruthTableCondition_6 && aVarTruthTableCondition_7 && ~aVarTruthTableCondition_8 && ~aVarTruthTableCondition_9 && ~aVarTruthTableCondition_11 && ~aVarTruthTableCondition_12)
    A6();
elseif (aVarTruthTableCondition_1 && ~aVarTruthTableCondition_2 && ~aVarTruthTableCondition_3 && aVarTruthTableCondition_6 && aVarTruthTableCondition_7 && aVarTruthTableCondition_9 && ~aVarTruthTableCondition_10 && ~aVarTruthTableCondition_11 && ~aVarTruthTableCondition_12)
    A2();
elseif (aVarTruthTableCondition_1 && ~aVarTruthTableCondition_2 && ~aVarTruthTableCondition_3 && aVarTruthTableCondition_6 && aVarTruthTableCondition_7 && aVarTruthTableCondition_8 && ~aVarTruthTableCondition_10 && ~aVarTruthTableCondition_11 && ~aVarTruthTableCondition_12)
    A3();
elseif (aVarTruthTableCondition_10 && ~aVarTruthTableCondition_11 && ~aVarTruthTableCondition_12)
    A7();
else % Default
    A1();
end

function A1()

% Aux Off

icmd_Base = fi(0,0,16,12);
icmd_Rod = fi(0,0,16,12);
state_AuxFlow = INACTIVE;

function A2()

% Aux Rod Full On

icmd_Base = fi(0,0,16,12);
icmd_Rod = icmd_Detent;
state_AuxFlow = ACTIVE;

function A3()

% Aux Base Full On

icmd_Base = icmd_Detent;
icmd_Rod = fi(0,0,16,12);
state_AuxFlow = ACTIVE;

function A4()

% Aux Rod Proportional

icmd_Base = fi(0,0,16,12);
icmd_Rod = icmd_Pot;
state_AuxFlow = ACTIVE;

function A5()

% Aux Base Proportional

icmd_Base = icmd_Pot;
icmd_Rod = fi(0,0,16,12);
state_AuxFlow = ACTIVE;

function A6()

% Reset ACD Variable Flow Flag

icmd_Base = fi(0,0,16,12);
icmd_Rod = fi(0,0,16,12);
ACDVarFlow = uint8(0);
state_AuxFlow = ACTIVE;

function A7()

% Inhibit ACD Command

icmd_Base = fi(0,0,16,12);
icmd_Rod = fi(0,0,16,12);
ACDVarFlow = ACDVarFlow - 1;
state_AuxFlow = ACTIVE;

== stateflow chart MX_Gtwy_Control/Inputs/SensorSupply2/Sensor_Supply2_State ==
  state NON_FUNCTIONAL
    en:state_SensorSupply2 = NORMAL;
  state OORH
    state Debounce
      en:debounceCount = 0;
      du:debounceCount++
    state OORH
      en:state_SensorSupply2 = OORH;
    (default) -> OORH.Debounce
    OORH.Debounce -> OORH.OORH : [(debounceCount >= CD_SENSOR_SUPPLY2_DEBOUNCE_OORH)...
        | || (state_SensorSupply2 == OORH)]
  state NORMAL
    state Debounce
      en:debounceCount = 0;
      du:debounceCount++
    state NORMAL
      en:state_SensorSupply2 = NORMAL;
    (default) -> NORMAL.Debounce
    NORMAL.Debounce -> NORMAL.NORMAL : [((debounceCount >= CD_SENSOR_SUPPLY2_DEBOUNCE_NORMAL)...
        | && (CD_SENSOR_SUPPLY2_DEBOUNCE_NORMAL != UNLIMITED))...
        | || (state_SensorSupply2 == NORMAL)]
  state OORL
    state Debounce
      en:debounceCount = 0;
      du:debounceCount++
    state NORMAL
      en:state_SensorSupply2 = OORL;
    (default) -> OORL.Debounce
    OORL.Debounce -> OORL.NORMAL : [(debounceCount >= CD_SENSOR_SUPPLY2_DEBOUNCE_OORL)...
        | || (state_SensorSupply2 == OORL)]
  (default) -> NON_FUNCTIONAL
  NON_FUNCTIONAL -> NORMAL : [(CD_SENSOR_SUPPLY2_FUNCTION != NON_FUNCTIONAL)]
  NORMAL -> OORH [order 2] : [(ain_SensorSupply2 >= CD_SENSOR_SUPPLY2_OORH)]
  NORMAL -> OORL [order 1] : [(ain_SensorSupply2 <= CD_SENSOR_SUPPLY2_OORL)...
      | && (calc_VBattery > CD_VBATTERY_LOW)]
  OORH -> NORMAL : [(ain_SensorSupply2 < CD_SENSOR_SUPPLY2_OORH)]
  OORL -> NORMAL : [(ain_SensorSupply2 > CD_SENSOR_SUPPLY2_OORL)...
      | || (calc_VBattery < CD_VBATTERY_LOW)]
  data: state_SensorSupply2 output uint8; ain_SensorSupply2 input uint16; CD_SENSOR_SUPPLY2_FUNCTION parameter uint8; CD_SENSOR_SUPPLY2_OORL parameter uint16; CD_SENSOR_SUPPLY2_OORH parameter uint16; CD_SENSOR_SUPPLY2_DEBOUNCE_NORMAL parameter uint16; CD_SENSOR_SUPPLY2_DEBOUNCE_OORH parameter uint16; CD_SENSOR_SUPPLY2_DEBOUNCE_OORL parameter uint16; debounceCount local uint16 =0; OORH parameter uint8; OORL parameter uint8; NON_FUNCTIONAL parameter uint8; NORMAL parameter uint8; UNLIMITED parameter uint16; calc_VBattery input fixdt(0,16,8); CD_VBATTERY_LOW parameter uint8

== stateflow chart MX_Gtwy_Control/Outputs/Dump_Valve/Dump_Valve/Dump_Valve ==
  state Off
    en:
    icmd_DumpValve = INACTIVE;
  state On
    en:
    icmd_DumpValve = CD_DUMP_VALVE_ON_CURRENT;
  (default) -> Off
  Off -> On : [aux_Flow == AUX_FLOW_MEDIUM]
  On -> Off : [aux_Flow != AUX_FLOW_MEDIUM]
  data: aux_Flow input uint8; INACTIVE parameter uint8; icmd_DumpValve output fixdt(0,16,12); CD_DUMP_VALVE_ON_CURRENT parameter fixdt(0,16,12); AUX_FLOW_MEDIUM parameter uint8

== stateflow chart MX_Gtwy_Control/Outputs/Hyd_Enable/Hyd_Enable/Hyd_Enable ==
  state Not_Cranking
    state Off
      en:
      dout_HydEnable = INACTIVE;
    state On
      en:
      dout_HydEnable = ACTIVE;
    (default) -> Off
    Off -> On : [state_Console == ACTIVE]
    On -> Off : [state_Console != ACTIVE]
  state Delay
  state Cranking
    en:
    dout_HydEnable = INACTIVE;
  (default) -> Not_Cranking
  Not_Cranking -> Cranking : [state_Engine == ENGINE_CRANKING]
  Delay -> Not_Cranking [order 1] : [after(CD_HYD_ENABLE_CRANK_DELAY, tick)]
  Delay -> Cranking [order 2] : [state_Engine == ENGINE_CRANKING]
  Cranking -> Delay : [state_Engine != ENGINE_CRANKING]
  data: state_Engine input uint8; state_Console input uint8; INACTIVE parameter uint8; ACTIVE parameter uint8; dout_HydEnable output boolean; ENGINE_CRANKING parameter uint8; CD_HYD_ENABLE_CRANK_DELAY parameter uint8

== stateflow chart MX_Gtwy_Control/Inputs/VBattery/LP_Filter_Adj_Gain/LP_Fitler_Adj_Gain ==
  state INITIAL
    en:Output = Input;
  state LP_FILTER
    du:Output = Output + ((Input - Output)/(1 << Gain));
  (default) -> INITIAL
  INITIAL -> LP_FILTER
  data: Gain input uint8; Input input fixdt(1,16,8); Output output fixdt(1,32,16)
